simulink model "CLRC_exp_A"  (R2023a)
settings: Solver=FixedStepDiscrete  StartTime=0.0  StopTime=inf  FixedStep=0.001  RelTol=1e-3  EnableMultiTasking=on
PreLoadFcn:
  | set_param(gcs, 'RTWSystemTargetFile', 'franka_emika_panda.tlc');
  | demos_common_configs();
values from neighbouring files (symbols this model uses):
  alpha = 3    [Panda_run.m]
  Kc = diag([20,20,20,20,8,8,5])    [Panda_run.m]
  Lambda = 10*eye(7)    [Panda_run.m]
  par = baseparmes()    [Panda_run.m]
  q0 = traj.qd.Data(:,:,1)    [Panda_run.m]
  Ts = 0.001    [Panda_run.m]
undefined symbols (not defined in the file or in its folder): DiscIntNeverNeededParam
libraries: simulink x7, franka_simulink_library x5
settings Model: SimulationMode=external  ExtModeTrigDuration=10000  Creator=vasi_ko  LastModifiedBy=stt
settings SolverCC: AlgebraicLoopSolver=TrustRegion
settings DataIOCC: LimitDataPoints=on  SaveFormat=Array  ReturnWorkspaceOutputs=off
settings OptimizationCC: DefaultParameterBehavior=Inlined  EnhancedBackFolding=on  PassReuseOutputArgsThreshold=0  OptimizeBlockOrder=speed  DifferentSizesBufferReuse=on  OptimizationCustomize=off
settings DebuggingCC: SolverPrmCheckMsg=warning  ParameterTunabilityLossMsg=error  UnconnectedInputMsg=warning  UnconnectedOutputMsg=warning  UnconnectedLineMsg=warning  SFUnexpectedBacktrackingDiag=warning  SFNoUnconditionalDefaultTransitionDiag=warning  SFExecutionAtInitializationDiag=none  ArithmeticOperatorsInVariantConditions=warning
settings HardwareCC: ProdBitPerLong=64  ProdHWDeviceType=Intel->x86-64 (Linux 64)
settings ModelReferenceCC: EnableRefExpFcnMdlSchedulingChecks=on  PropagateSignalLabelsOutOfModel=off
settings SFSimCC: SimUseLocalCustomCode=off  MATLABDynamicMemAlloc=off  CustomCodeUndefinedFunction=UseInterfaceOnly  SimTargetLang=C++
settings RTWCC: SystemTargetFile=franka_emika_panda.tlc  RTWVerbose=off  RTWUseLocalCustomCode=off  Toolchain=Franka Simulink | gmake (64-bit linux)  BuildConfiguration=Faster Runs  TargetLang=C++  DLArmComputeVersion=19.05
settings CodeAppCC: ForceParamTrailComments=on  OperatorAnnotations=on
settings STFCustomTargetCC: ModelReferenceCompliant=off  ConcurrentExecutionCompliant=off  MatFileLogging=on  SystemTargetFile=franka_emika_panda.tlc
settings GRTTargetCC: ModelReferenceCompliant=off  ParMdlRefBuildCompliant=off  CompOptLevelCompliant=off  ConcurrentExecutionCompliant=off  CombineOutputUpdateFcns=off  CPPClassGenCompliant=off  GRTInterface=on  ExtModeStaticAlloc=on  ExtModeTransport=1  ExtModeMexFile=ext_xcp  ExtModeIntrfLevel=Level2 - Open
settings ConfigComp: CovMetricSettings=dw  CovSaveCumulativeToWorkspaceVar=on  CovSaveSingleToWorkspaceVar=on  CovExternalEMLEnable=off  CovSFcnEnable=off

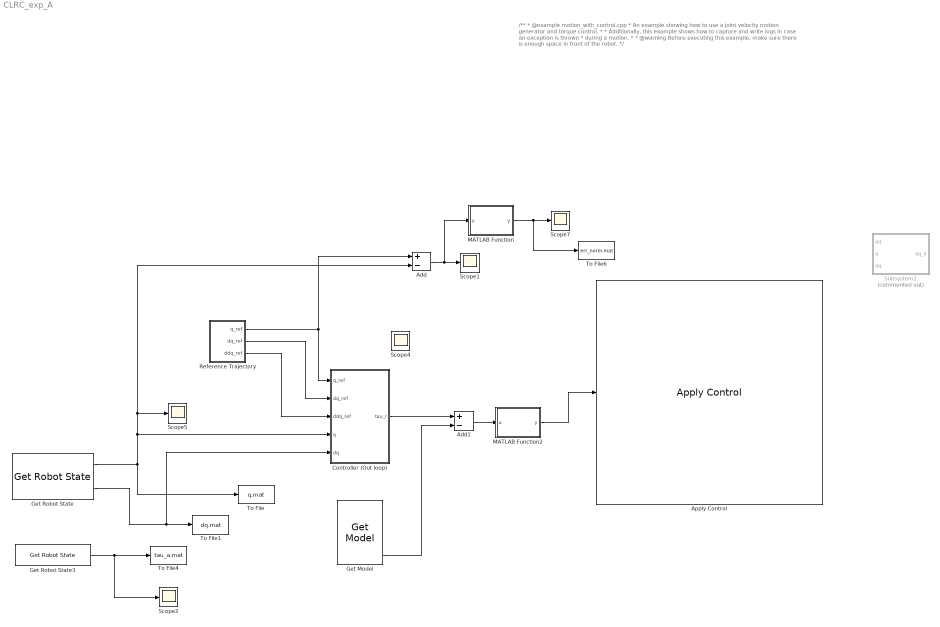
== system CLRC_exp_A ==
  note: /**
      |  * @example motion_with_control.cpp
      |  * An example showing how to use a joint velocity motion generator and torque control.
      |  *
      |  * Additionally, this example shows how to capture and write logs in case an exception is thrown
      |  * during a motion.
      |  *
      |  * @warning Before executing this example, make sure there is enough space in front of the robot.
      |  */
  Add : Sum  Inputs=+-
  Add1 : Sum  Inputs=+-
  Apply Control : franka_simulink_library/Apply Control  robot_ip=robot_ip  gripper_attached=off  control_mode=Torque Control  controller_choice=Joint Impedance Controller  elbow_control=on  collision_thresholds=collision_behavior  joint_impedance=joint_impedance  cartesian_impedance=cartesian_impedance  load_inertia=load_inertia  EE_T_K=EE_T_K  init_joint_configuration_req=on  init_joint_configuration=q0'
  Controller (Out loop) : Subsystem
  Get Model : franka_simulink_library/Get Model  robot_ip=robot_ip
  Get Robot State : franka_simulink_library/Get Robot State  robot_ip=robot_ip  signals_requested='q dq'
  Get Robot State3 : franka_simulink_library/Get Robot State  robot_ip=robot_ip  signals_requested='tau_J'
  MATLAB Function : MATLAB Function  TreatAsAtomicUnit=on
  MATLAB Function2 : MATLAB Function  TreatAsAtomicUnit=on
  Reference Trajectory : Subsystem
  Scope{1..2,4..5,7} (5 alike) : Scope
  Subsystem2 : Subsystem  Commented=on
  To File : ToFile  Filename=q.mat  MatrixName=q
  To File1 : ToFile  Filename=dq.mat  MatrixName=dq
  To File4 : ToFile  Filename=tau_a.mat  MatrixName=tau_a
  To File6 : ToFile  Filename=err_norm.mat  MatrixName=err_norm
  wires:
  Get Robot State3/1 -> Scope2/1, To File4/1
  Add/1 -> MATLAB Function/1(u), Scope1/1
  Get Robot State/2 -> Controller (Out loop)/5(dq), To File1/1
  MATLAB Function/1(y) -> To File6/1, Scope7/1
  Get Robot State/1 -> Controller (Out loop)/4(q), Scope5/1, Add/2, To File/1
  MATLAB Function2/1(y) -> Apply Control/1
  Reference Trajectory/1(q_ref) -> Controller (Out loop)/1(q_ref), Add/1
  Reference Trajectory/2(dq_ref) -> Controller (Out loop)/2(dq_ref)
  Reference Trajectory/3(ddq_ref) -> Controller (Out loop)/3(ddq_ref)
  Controller (Out loop)/1(tau_r) -> Add1/1
  Add1/1 -> MATLAB Function2/1(u)
  Get Model/6 -> Add1/2

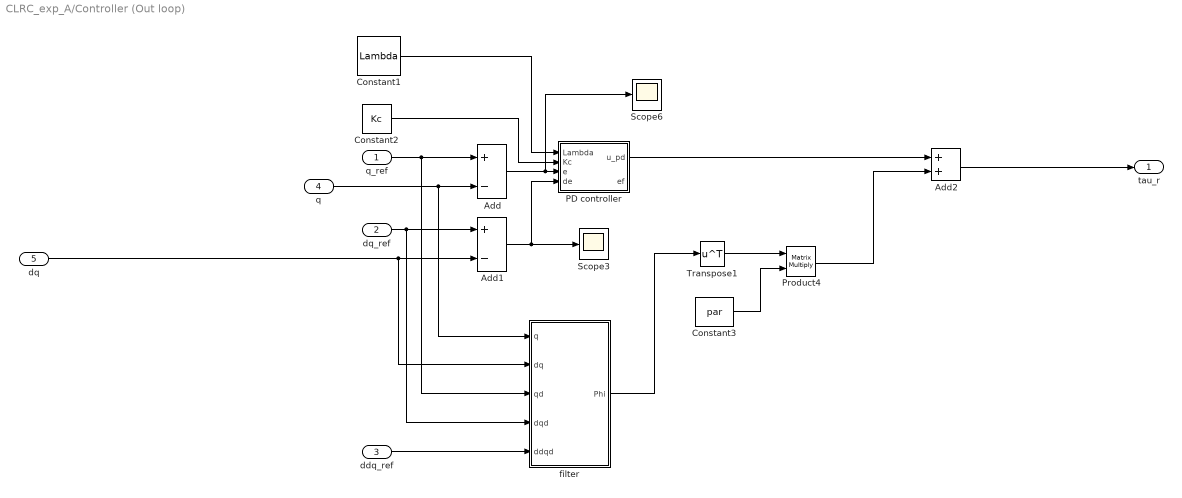
== system CLRC_exp_A/Controller (Out loop) ==
  q_ref : Inport
  dq_ref : Inport  Port=2
  ddq_ref : Inport  Port=3
  q : Inport  Port=4
  dq : Inport  Port=5
  Add : Sum  Inputs=+-
  Add1 : Sum  Inputs=+-
  Add2 : Sum
  Constant1 : Constant  Value=Lambda
  Constant2 : Constant  Value=Kc
  Constant3 : Constant  Value=par
  PD controller : MATLAB Function  TreatAsAtomicUnit=on
  Product4 : Product  Multiplication=Matrix(*)
  Scope3 : Scope
  Scope6 : Scope
  Transpose1 : Math  Operator=transpose
  filter : Subsystem
  tau_r : Outport
  wires:
  q_ref/1 -> filter/3(qd), Add/1
  dq_ref/1 -> filter/4(dqd), Add1/1
  Add/1 -> Scope6/1, PD controller/3(e)
  Constant2/1 -> PD controller/2(Kc)
  Constant1/1 -> PD controller/1(Lambda)
  Add1/1 -> PD controller/4(de), Scope3/1
  ddq_ref/1 -> filter/5(ddqd)
  Add2/1 -> tau_r/1
  q/1 -> filter/1(q), Add/2
  dq/1 -> Add1/2, filter/2(dq)
  PD controller/1(u_pd) -> Add2/1
  Transpose1/1 -> Product4/1
  filter/1(Phi) -> Transpose1/1
  Constant3/1 -> Product4/2
  Product4/1 -> Add2/2

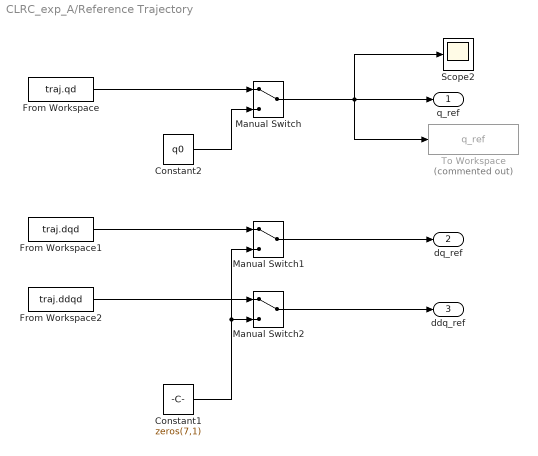
== system CLRC_exp_A/Reference Trajectory ==
  Constant1 : Constant  Value=zeros(7,1)
  Constant2 : Constant  Value=q0
  From Workspace : FromWorkspace  VariableName=traj.qd
  From Workspace1 : FromWorkspace  VariableName=traj.dqd
  From Workspace2 : FromWorkspace  VariableName=traj.ddqd
  Manual Switch : ManualSwitch
  Manual Switch1 : ManualSwitch
  Manual Switch2 : ManualSwitch
  Scope2 : Scope
  To Workspace : ToWorkspace  Commented=on  VariableName=q_ref  SaveFormat=Timeseries  SampleTime=-1
  q_ref : Outport
  dq_ref : Outport  Port=2
  ddq_ref : Outport  Port=3
  wires:
  Constant2/1 -> Manual Switch/2
  Manual Switch/1 -> To Workspace/1, Scope2/1, q_ref/1
  Manual Switch1/1 -> dq_ref/1
  Manual Switch2/1 -> ddq_ref/1
  Constant1/1 -> Manual Switch1/2, Manual Switch2/2
  From Workspace/1 -> Manual Switch/1
  From Workspace1/1 -> Manual Switch1/1
  From Workspace2/1 -> Manual Switch2/1

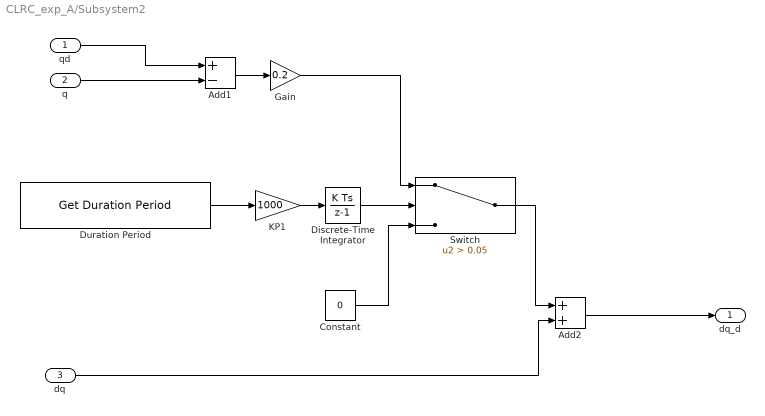
== system CLRC_exp_A/Subsystem2 ==
  qd : Inport
  q : Inport  Port=2
  dq : Inport  Port=3
  Add1 : Sum  Inputs=+-
  Add2 : Sum
  Constant : Constant  Value=0
  Discrete-Time Integrator : DiscreteIntegrator  SampleTime=-1  ICPrevOutput=DiscIntNeverNeededParam  ICPrevScaledInput=DiscIntNeverNeededParam
  Duration Period : franka_simulink_library/Get Duration Period  robot_ip=robot_ip
  Gain : Gain  Gain=0.2
  KP1 : Gain  Gain=1000
  Switch : Switch  Criteria=u2 > Threshold  Threshold=0.05  SaturateOnIntegerOverflow=off  InputSameDT=off
  dq_d : Outport
  wires:
  Add1/1 -> Gain/1
  qd/1 -> Add1/1
  q/1 -> Add1/2
  dq/1 -> Add2/2
  Add2/1 -> dq_d/1
  Duration Period/1 -> KP1/1
  KP1/1 -> Discrete-Time Integrator/1
  Discrete-Time Integrator/1 -> Switch/2
  Gain/1 -> Switch/1
  Switch/1 -> Add2/1
  Constant/1 -> Switch/3

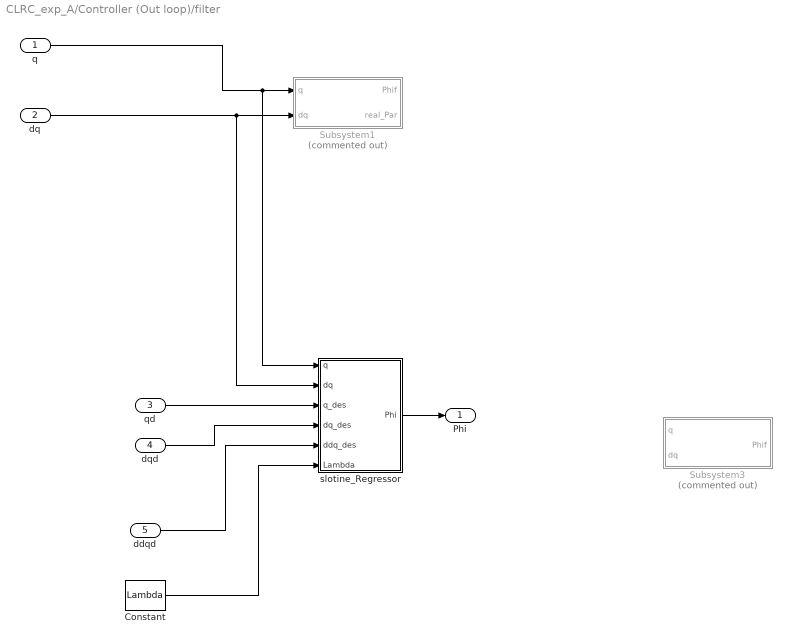
== system CLRC_exp_A/Controller (Out loop)/filter ==
  q : Inport
  dq : Inport  Port=2
  qd : Inport  Port=3
  dqd : Inport  Port=4
  ddqd : Inport  Port=5
  Constant : Constant  Value=Lambda
  Subsystem1 : Subsystem  Commented=on
  Subsystem3 : Subsystem  Commented=on
  slotine_Regressor : MATLAB Function  TreatAsAtomicUnit=on
  Phi : Outport
  wires:
  qd/1 -> slotine_Regressor/3(q_des)
  dqd/1 -> slotine_Regressor/4(dq_des)
  ddqd/1 -> slotine_Regressor/5(ddq_des)
  Constant/1 -> slotine_Regressor/6(Lambda)
  q/1 -> Subsystem1/1(q), slotine_Regressor/1(q)
  slotine_Regressor/1(Phi) -> Phi/1
  dq/1 -> Subsystem1/2(dq), slotine_Regressor/2(dq)

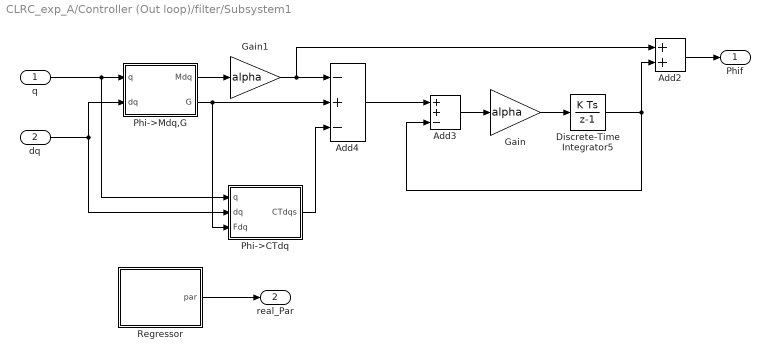
== system CLRC_exp_A/Controller (Out loop)/filter/Subsystem1 ==
  q : Inport
  dq : Inport  Port=2
  Add2 : Sum
  Add3 : Sum  Inputs=++-
  Add4 : Sum  Inputs=-+-
  Discrete-Time Integrator5 : DiscreteIntegrator  SampleTime=-1  ICPrevOutput=DiscIntNeverNeededParam  ICPrevScaledInput=DiscIntNeverNeededParam
  Gain : Gain  Gain=alpha
  Gain1 : Gain  Gain=alpha
  Phi->CTdq : MATLAB Function  TreatAsAtomicUnit=on
  Phi->Mdq,G : MATLAB Function  TreatAsAtomicUnit=on
  Regressor : MATLAB Function  TreatAsAtomicUnit=on
  Phif : Outport
  real_Par : Outport  Port=2
  wires:
  Add4/1 -> Add3/1
  Add2/1 -> Phif/1
  Discrete-Time Integrator5/1 -> Add2/2, Add3/3
  Add3/1 -> Gain/1
  Gain/1 -> Discrete-Time Integrator5/1
  Regressor/1(par) -> real_Par/1
  q/1 -> Phi->CTdq/1(q), Phi->Mdq,G/1(q)
  dq/1 -> Phi->CTdq/2(dq), Phi->Mdq,G/2(dq)
  Phi->Mdq,G/1(Mdq) -> Gain1/1
  Gain1/1 -> Add2/1, Add4/1
  Phi->Mdq,G/2(G) -> Add4/2, Phi->CTdq/3(Fdq)
  Phi->CTdq/1(CTdqs) -> Add4/3

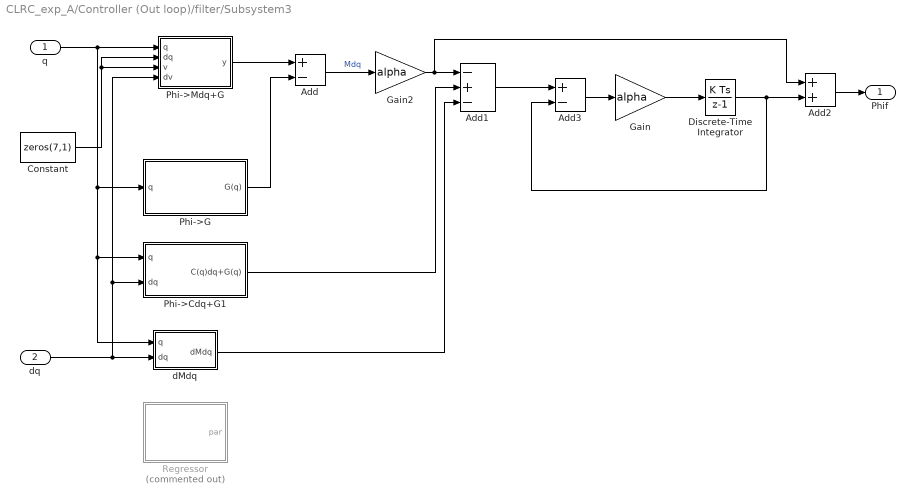
== system CLRC_exp_A/Controller (Out loop)/filter/Subsystem3 ==
  q : Inport
  dq : Inport  Port=2
  Add : Sum  Inputs=+-
  Add1 : Sum  Inputs=-+-
  Add2 : Sum
  Add3 : Sum  Inputs=+-
  Constant : Constant  Value=zeros(7,1)
  Discrete-Time Integrator : DiscreteIntegrator  SampleTime=Ts  ICPrevOutput=DiscIntNeverNeededParam  ICPrevScaledInput=DiscIntNeverNeededParam
  Gain : Gain  Gain=alpha
  Gain2 : Gain  Gain=alpha
  Phi->Cdq+G1 : Subsystem
  Phi->G : Subsystem
  Phi->Mdq+G : MATLAB Function  TreatAsAtomicUnit=on
  Regressor : MATLAB Function  Commented=on  TreatAsAtomicUnit=on
  dMdq : Subsystem
  Phif : Outport
  wires:
  Constant/1 -> Phi->Mdq+G/3(v), Phi->Mdq+G/2(dq)
  q/1 -> Phi->G/1(q), Phi->Cdq+G1/1(q), dMdq/1(q), Phi->Mdq+G/1(q)
  dq/1 -> Phi->Mdq+G/4(dv), Phi->Cdq+G1/2(dq), dMdq/2(dq)
  Phi->Mdq+G/1(y) -> Add/1
  Phi->G/1(G(q)) -> Add/2
  Add/1 -> Gain2/1  "Mdq"
  Add1/1 -> Add3/1
  Add2/1 -> Phif/1
  Phi->Cdq+G1/1(C(q)dq+G(q)) -> Add1/2
  Discrete-Time Integrator/1 -> Add3/2, Add2/2
  Gain2/1 -> Add2/1, Add1/1
  Add3/1 -> Gain/1
  Gain/1 -> Discrete-Time Integrator/1
  dMdq/1(dMdq) -> Add1/3

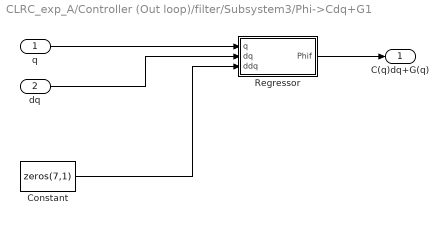
== system CLRC_exp_A/Controller (Out loop)/filter/Subsystem3/Phi->Cdq+G1 ==
  q : Inport
  dq : Inport  Port=2
  Constant : Constant  Value=zeros(7,1)
  Regressor : MATLAB Function  TreatAsAtomicUnit=on
  C(q)dq+G(q) : Outport
  wires:
  q/1 -> Regressor/1(q)
  Constant/1 -> Regressor/3(ddq)
  Regressor/1(Phif) -> C(q)dq+G(q)/1
  dq/1 -> Regressor/2(dq)

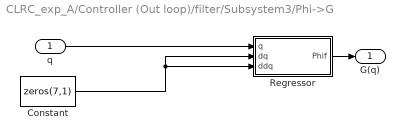
== system CLRC_exp_A/Controller (Out loop)/filter/Subsystem3/Phi->G ==
  q : Inport
  Constant : Constant  Value=zeros(7,1)
  Regressor : MATLAB Function  TreatAsAtomicUnit=on
  G(q) : Outport
  wires:
  q/1 -> Regressor/1(q)
  Constant/1 -> Regressor/3(ddq), Regressor/2(dq)
  Regressor/1(Phif) -> G(q)/1

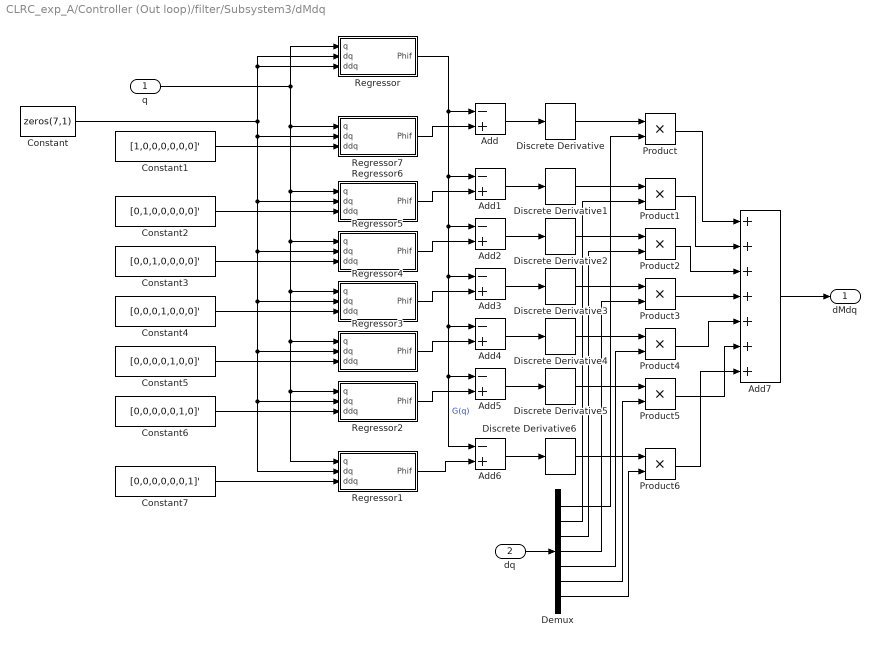
== system CLRC_exp_A/Controller (Out loop)/filter/Subsystem3/dMdq ==
  q : Inport
  dq : Inport  Port=2
  Add{,1..6} (7 alike) : Sum  Inputs=-+
  Add7 : Sum  Inputs=+++++++
  Constant : Constant  Value=zeros(7,1)
  Constant1 : Constant  Value=[1,0,0,0,0,0,0]'
  Constant2 : Constant  Value=[0,1,0,0,0,0,0]'
  Constant3 : Constant  Value=[0,0,1,0,0,0,0]'
  Constant4 : Constant  Value=[0,0,0,1,0,0,0]'
  Constant5 : Constant  Value=[0,0,0,0,1,0,0]'
  Constant6 : Constant  Value=[0,0,0,0,0,1,0]'
  Constant7 : Constant  Value=[0,0,0,0,0,0,1]'
  Demux : Demux  Outputs=7
  Discrete Derivative{,1..6} (7 alike) : simulink/Discrete/Discrete Derivative  gainval=1.0  ICPrevScaledInput=0.0  OutMin=[]  OutMax=[]  OutDataType=fixdt(1, 16)  OutScaling=2^-10  DoSatur=off
  Product{,1..6} (7 alike) : Product
  Regressor : MATLAB Function  TreatAsAtomicUnit=on
  Regressor1 : MATLAB Function  TreatAsAtomicUnit=on
  Regressor2 : MATLAB Function  TreatAsAtomicUnit=on
  Regressor3 : MATLAB Function  TreatAsAtomicUnit=on
  Regressor4 : MATLAB Function  TreatAsAtomicUnit=on
  Regressor5 : MATLAB Function  TreatAsAtomicUnit=on
  Regressor6 : MATLAB Function  TreatAsAtomicUnit=on
  Regressor7 : MATLAB Function  TreatAsAtomicUnit=on
  dMdq : Outport
  wires:
  q/1 -> Regressor/1(q), Regressor1/1(q), Regressor2/1(q), Regressor3/1(q), Regressor4/1(q), Regressor5/1(q), Regressor6/1(q), Regressor7/1(q)
  Constant/1 -> Regressor/2(dq), Regressor/3(ddq), Regressor1/2(dq), Regressor2/2(dq), Regressor3/2(dq), Regressor4/2(dq), Regressor5/2(dq), Regressor6/2(dq), Regressor7/2(dq)
  Constant2/1 -> Regressor6/3(ddq)
  Constant1/1 -> Regressor7/3(ddq)
  Constant3/1 -> Regressor5/3(ddq)
  Constant4/1 -> Regressor4/3(ddq)
  Constant5/1 -> Regressor3/3(ddq)
  Constant6/1 -> Regressor2/3(ddq)
  Constant7/1 -> Regressor1/3(ddq)
  Regressor7/1(Phif) -> Add/2
  Regressor/1(Phif) -> Add1/1, Add2/1, Add3/1, Add4/1, Add5/1, Add6/1, Add/1  "G(q)"
  Regressor5/1(Phif) -> Add2/2
  Regressor6/1(Phif) -> Add1/2
  Regressor4/1(Phif) -> Add3/2
  Regressor3/1(Phif) -> Add4/2
  Regressor2/1(Phif) -> Add5/2
  Regressor1/1(Phif) -> Add6/2
  Add/1 -> Discrete Derivative/1
  Add1/1 -> Discrete Derivative1/1
  Add2/1 -> Discrete Derivative2/1
  Add3/1 -> Discrete Derivative3/1
  Add5/1 -> Discrete Derivative5/1
  Add6/1 -> Discrete Derivative6/1
  Add4/1 -> Discrete Derivative4/1
  dq/1 -> Demux/1
  Discrete Derivative/1 -> Product/1
  Demux/1 -> Product/2
  Discrete Derivative1/1 -> Product1/1
  Discrete Derivative2/1 -> Product2/1
  Discrete Derivative3/1 -> Product3/1
  Discrete Derivative4/1 -> Product4/1
  Discrete Derivative5/1 -> Product5/1
  Discrete Derivative6/1 -> Product6/1
  Demux/7 -> Product6/2
  Demux/6 -> Product5/2
  Demux/5 -> Product4/2
  Demux/4 -> Product3/2
  Demux/3 -> Product2/2
  Demux/2 -> Product1/2
  Product/1 -> Add7/1
  Product1/1 -> Add7/2
  Product2/1 -> Add7/3
  Product3/1 -> Add7/4
  Product4/1 -> Add7/5
  Product5/1 -> Add7/6
  Product6/1 -> Add7/7
  Add7/1 -> dMdq/1

== matlab function CLRC_exp_A/Controller (Out loop)/filter/Subsystem3/dMdq/Regressor2 ==
  data: q input; Phif output; dq input; ddq input
function Phif= fcn(q,dq,ddq)
Phif=Base_Regressor_Panda(q,dq,ddq);

== matlab function CLRC_exp_A/Controller (Out loop)/filter/Subsystem3/dMdq/Regressor3 ==
  data: q input; Phif output; dq input; ddq input
function Phif= fcn(q,dq,ddq)
Phif=Base_Regressor_Panda(q,dq,ddq);

== matlab function CLRC_exp_A/Controller (Out loop)/filter/Subsystem3/dMdq/Regressor4 ==
  data: q input; Phif output; dq input; ddq input
function Phif= fcn(q,dq,ddq)
Phif=Base_Regressor_Panda(q,dq,ddq);

== matlab function CLRC_exp_A/Controller (Out loop)/PD controller ==
  data: Lambda input; Kc input; e input; u_pd output; de input; ef output
function [u_pd,ef]= fcn(Lambda,Kc,e,de)

ef=de+Lambda*e;

u_pd = Kc*ef;

== matlab function CLRC_exp_A/Controller (Out loop)/filter/Subsystem3/Regressor ==
  data: par output
function par= fcn()

par=baseparmes();

== matlab function CLRC_exp_A/Controller (Out loop)/filter/Subsystem3/dMdq/Regressor ==
  data: q input; Phif output; dq input; ddq input
function Phif= fcn(q,dq,ddq)
Phif=Base_Regressor_Panda(q,dq,ddq);

== matlab function CLRC_exp_A/Controller (Out loop)/filter/Subsystem3/dMdq/Regressor5 ==
  data: q input; Phif output; dq input; ddq input
function Phif= fcn(q,dq,ddq)
Phif=Base_Regressor_Panda(q,dq,ddq);

== matlab function CLRC_exp_A/Controller (Out loop)/filter/Subsystem3/dMdq/Regressor6 ==
  data: q input; Phif output; dq input; ddq input
function Phif= fcn(q,dq,ddq)
Phif=Base_Regressor_Panda(q,dq,ddq);

== matlab function CLRC_exp_A/Controller (Out loop)/filter/Subsystem3/dMdq/Regressor7 ==
  data: q input; Phif output; dq input; ddq input
function Phif= fcn(q,dq,ddq)
Phif=Base_Regressor_Panda(q,dq,ddq);

== matlab function CLRC_exp_A/Controller (Out loop)/filter/Subsystem3/Phi->Mdq+G ==
  data: q input; y output; dq input; v input; dv input
function y = fcn(q,dq,v,dv)

%y = min(svd(u));
y=Base_Slotine_Panda(q,dq,v,dv);

== matlab function CLRC_exp_A/MATLAB Function ==
  data: u input; y output
function y = fcn(u)

y = norm(u);

== matlab function CLRC_exp_A/MATLAB Function2 ==
  data: u input; y output
function y = fcn(u)

y = u';

== matlab function CLRC_exp_A/Controller (Out loop)/filter/slotine_Regressor ==
  data: q input; Phi output; dq input; q_des input; dq_des input; ddq_des input; Lambda input
function Phi = fcn(q,dq,q_des,dq_des,ddq_des,Lambda)
e1=q-q_des;
de1=dq-dq_des;
dqr=dq_des-Lambda*e1;
ddqr=ddq_des-Lambda*de1;

Phi=Base_Slotine_Panda(q,dq,dqr,ddqr);

== matlab function CLRC_exp_A/Controller (Out loop)/filter/Subsystem1/Phi->CTdq ==
  data: q input; CTdqs output; dq input; Fdq input
function CTdqs = fcn(q,dq,Fdq)

CTdqs=Coriolis_Reg(q,dq,Fdq);
% I=eye(7);
% CTdqs=zeros(7,64)';
% for k=1:7
%     CorReg=Coriolis_Reg(q,dq,I(:,k));
%     for i=1:7
%         CTdq(k,:)=CTdq(k,:)+dq(i)*CorReg(i,:);
%     end
% end
% CTdqs=CTdq';


% CTdqs=[Coriolis_Reg(q,dq,I(:,1))'*dq,Coriolis_Reg(q,dq,I(:,2))'*dq,Coriolis_Reg(q,dq,I(:,3))'*dq,...
%     Coriolis_Reg(q,dq,I(:,4))'*dq,Coriolis_Reg(q,dq,I(:,5))'*dq,Coriolis_Reg(q,dq,I(:,6))'*dq,...
%     Coriolis_Reg(q,dq,I(:,7))'*dq];

== matlab function CLRC_exp_A/Controller (Out loop)/filter/Subsystem1/Phi->Mdq,G ==
  data: q input; Mdq output; dq input; G output
function [Mdq,G] = fcn(q,dq)

%y = min(svd(u));
vec0=zeros(7,1);
%Fdq=Base_Slotine_Panda(q,dq,vec0,vec0)-Base_Regressor_Panda(q,vec0,vec0);
G=Base_Slotine_Panda(q,dq,vec0,vec0);
%Mdq=Base_Slotine_Panda(q,vec0,vec0,dq)-Base_Regressor_Panda(q,vec0,vec0);
Mdq=Base_Regressor_Panda(q,vec0,dq)-Base_Regressor_Panda(q,vec0,vec0);

== matlab function CLRC_exp_A/Controller (Out loop)/filter/Subsystem1/Regressor ==
  data: par output
function par= fcn()

par=baseparmes();

== matlab function CLRC_exp_A/Controller (Out loop)/filter/Subsystem3/Phi->Cdq+G1/Regressor ==
  data: q input; Phif output; dq input; ddq input
function Phif= fcn(q,dq,ddq)
Phif=Base_Regressor_Panda(q,dq,ddq);

== matlab function CLRC_exp_A/Controller (Out loop)/filter/Subsystem3/Phi->G/Regressor ==
  data: q input; Phif output; dq input; ddq input
function Phif= fcn(q,dq,ddq)
Phif=Base_Regressor_Panda(q,dq,ddq);

== matlab function CLRC_exp_A/Controller (Out loop)/filter/Subsystem3/dMdq/Regressor1 ==
  data: q input; Phif output; dq input; ddq input
function Phif= fcn(q,dq,ddq)
Phif=Base_Regressor_Panda(q,dq,ddq);
